simulink model "BaseEngineController_A02"  (Simulink 7.7)
settings: Solver=FixedStepDiscrete  StartTime=0.0  StopTime=10.0  RelTol=1e-3
PreLoadFcn:
  | try, eval([bdroot '_setup']); catch, eval('model_setup', ''); end
InitFcn:
  | try, eval([bdroot '_setup']); catch, eval('model_setup', ''); end
undefined symbols (not defined in the file or in its folder): ECU555, High, motohawk_build_version, motohawk_trunc_name, motohawk_vardec_config_group, motohawk_vardec_path, True
libraries: MotoHawk_lib x1041, AutoDoc_lib x697, motohawk_control_primitives_lib x106, motohawk_engine_lib x18, motohawk_annotations_lib x12, simulink x9, motohawk_control_primitives_motoshare_lib x1, simulink_extras x1
(part 3 of 21)

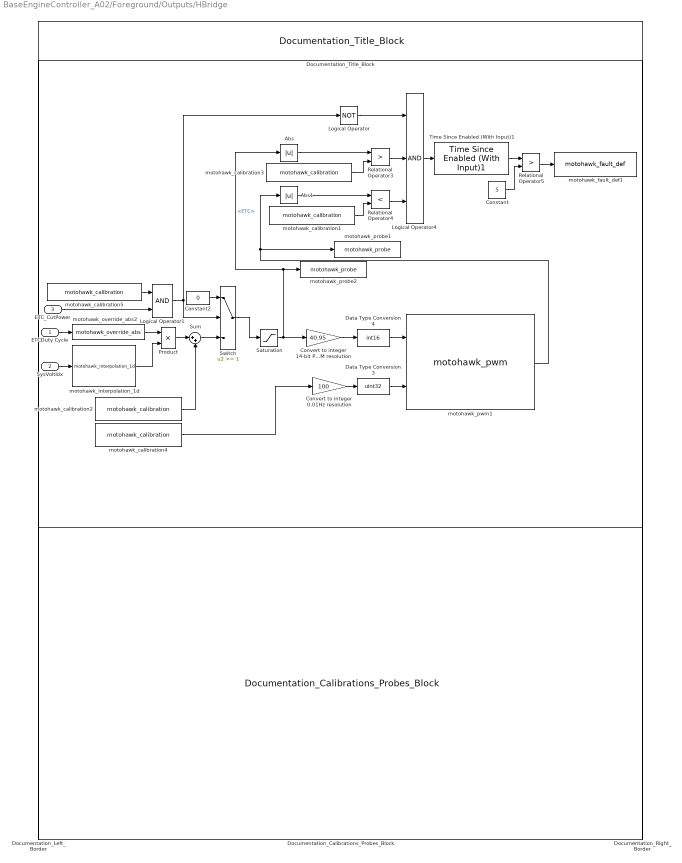
== system BaseEngineController_A02/Foreground/Outputs/HBridge ==
  ETCDuty Cycle : Inport
  SysVoltIdx : Inport  Port=2
  ETC_CutPower : Inport  Port=3
  Abs : Abs
  Abs1 : Abs
  Constant : Constant  Value=5
  Constant2 : Constant  Value=0  SampleTime=-1
  Convert to integer 0.01Hz resolution : Gain  Gain=100  SaturateOnIntegerOverflow=on  OutDataTypeStr=Inherit: Same as input  ParamDataTypeStr=Inherit: Same as input
  Convert to integer 14-bit PWM resolution : Gain  Gain=40.95  SaturateOnIntegerOverflow=on  OutDataTypeStr=Inherit: Same as input  ParamDataTypeStr=Inherit: Same as input
  Data Type Conversion3 : DataTypeConversion  OutDataTypeStr=uint32  SaturateOnIntegerOverflow=on  RndMeth=Zero
  Data Type Conversion4 : DataTypeConversion  OutDataTypeStr=int16  SaturateOnIntegerOverflow=on  RndMeth=Zero
  Documentation_Calibrations_Probes_Block : AutoDoc_lib/Documentation_Calibrations_Probes_Block
  Documentation_Left_Border : AutoDoc_lib/Documentation_Left_Border  layout=Portrait
  Documentation_Right_Border : AutoDoc_lib/Documentation_Right_Border  layout=Portrait
  Documentation_Title_Block : AutoDoc_lib/Documentation_Title_Block
  Logical Operator : Logic  Operator=NOT  AllPortsSameDT=off  OutDataTypeStr=boolean
  Logical Operator1 : Logic  AllPortsSameDT=off  OutDataTypeStr=boolean
  Logical Operator4 : Logic  Inputs=3  AllPortsSameDT=off  OutDataTypeStr=boolean
  Product : Product  SaturateOnIntegerOverflow=on  InputSameDT=on  OutDataTypeStr=Inherit: Same as first input
  Relational Operator3 : RelationalOperator  Operator=>  OutDataTypeStr=boolean
  Relational Operator4 : RelationalOperator  Operator=<  OutDataTypeStr=boolean
  Relational Operator5 : RelationalOperator  Operator=>  OutDataTypeStr=boolean
  Saturation : Saturate  UpperLimit=100  LowerLimit=-100
  Sum : Sum  Inputs=|++  SaturateOnIntegerOverflow=on
  Switch : Switch  Threshold=1
  Time Since Enabled (With Input)1 : motohawk_control_primitives_lib/Time Since Enabled (With Input)1  SystemSampleTime=-1  nam='ETCOpenFaultTimer'  samp_time=-1  init=0  upperlim=16000
  motohawk_calibration1 : MotoHawk_lib/Calibration & Probing Blocks/motohawk_calibration  nam='ETC_I_MIN'  val=5  storage=Calibration  show_more=on  name_source=Use Parameter  data_type=double  read_level=1  write_level=1  ovr_cals=on  view_as=Number  enum_struct={'Disabled', 'Enabled'}  vector_style=Wide Row  use_help_units=on  help="'H-bridge open circuit fault:  ETC current must exceed this when ETC duty cycle is > ETC_OC_MIN, otherwise an open circuit fault may exist.'"  units='mA'  use_header_enum=off  row_header_enum={}  col_header_enum={}  use_min_max=off  min_val=0  max_val=inf  use_precision=off  precision=''  gain=1  offset=0  exp=1  use_mototune_group=on  mototune_group=motohawk_vardec_path(gcb)
  motohawk_calibration2 : MotoHawk_lib/Calibration & Probing Blocks/motohawk_calibration  nam='ETC_Offset'  val=0  storage=Calibration  show_more=on  name_source=Use Parameter  data_type=double  read_level=1  write_level=1  ovr_cals=on  view_as=Number  enum_struct={'Disabled', 'Enabled'}  vector_style=Wide Row  use_help_units=on  help='Offset from engineering units to PWM duty cycle'  units='%'  use_header_enum=off  row_header_enum={}  col_header_enum={}  use_min_max=off  min_val=-inf  max_val=inf  use_precision=off  precision=''  gain=1  offset=0  exp=1  use_mototune_group=on  mototune_group=motohawk_vardec_path(gcb)
  motohawk_calibration3 : MotoHawk_lib/Calibration & Probing Blocks/motohawk_calibration  nam='ETC_OC_MIN'  val=50  storage=Calibration  show_more=on  name_source=Use Parameter  data_type=double  read_level=1  write_level=1  ovr_cals=on  view_as=Number  enum_struct={'Disabled', 'Enabled'}  vector_style=Wide Row  use_help_units=on  help="'H-bridge open circuit fault:  ETC duty cycle must exceed this to enable open circuit fault detection.'"  units='%'  use_header_enum=off  row_header_enum={}  col_header_enum={}  use_min_max=off  min_val=0  max_val=inf  use_precision=off  precision=''  gain=1  offset=0  exp=1  use_mototune_group=on  mototune_group=motohawk_vardec_path(gcb)
  motohawk_calibration4 : MotoHawk_lib/Calibration & Probing Blocks/motohawk_calibration  nam='ETC_Frequency'  val=2500  storage=Calibration  show_more=on  name_source=Use Parameter  data_type=double  read_level=1  write_level=1  ovr_cals=on  view_as=Number  enum_struct={'Disabled', 'Enabled'}  vector_style=Wide Row  use_help_units=on  help='Frequency of PWM output'  units='Hz'  use_header_enum=off  row_header_enum={}  col_header_enum={}  use_min_max=on  min_val=7.63  max_val=inf  use_precision=off  precision=''  gain=1  offset=0  exp=1  use_mototune_group=on  mototune_group=motohawk_vardec_path(gcb)
  motohawk_calibration5 : MotoHawk_lib/Calibration & Probing Blocks/motohawk_calibration  nam='ETC_CutPower_Permitted'  val=1  storage=Calibration  show_more=on  name_source=Use Parameter  data_type=boolean  read_level=1  write_level=1  ovr_cals=on  view_as=Number  enum_struct={'Disabled', 'Enabled'}  vector_style=Wide Row  use_help_units=on  help='Cut power to ETC after ESTOP or long stalled period'  units='flag'  use_header_enum=off  row_header_enum={}  col_header_enum={}  use_min_max=off  min_val=0  max_val=100  use_precision=on  precision='2.3'  gain=1  offset=0  exp=1  use_mototune_group=on  mototune_group=motohawk_vardec_path(gcb)
  motohawk_fault_def1 : MotoHawk_lib/Fault Management Blocks/motohawk_fault_def  nam='ETC_Open_Fault'  mode=Enabled  id=[]  x=25  y=50  input_suspected=on  indeterminate=off  increment_xy=on  downsample_count=1  ovr_cals=on  ovr_actions=on  show_actions=off  action1_name=''  action1_cond=(None)  action2_name=''  action2_cond=(None)  action3_name=''  action3_cond=(None)  action4_name=''  action4_cond=(None)
  motohawk_interpolation_1d : MotoHawk_lib/Lookup Tables/motohawk_interpolation_1d  nam='ETC_VoltageComp'  table_data=ones(1,9)  show_more=on  data_type=Inherit from 'Table Data'  storage=NonAdaptive  read_level=1  write_level=1  ovr_cals=on  use_help=on  help='Gain on ETC duty cycle based on DRVP'  units=''  use_min_max=off  min_val=-inf  max_val=inf  use_precision=off  precision=''  gain=1  offset=0  exp=1  use_links=off  prelookup_block=''  output_name=''  use_mototune_group=on  mototune_group=motohawk_vardec_path(gcb)
  motohawk_override_abs2 : MotoHawk_lib/Calibration & Probing Blocks/motohawk_override_abs  SystemSampleTime=-1  parent_name='ETC'  parent_val=0  show_more=on  name_source=Use Parameter  parent_read_level=1  parent_override_level=1  view_as=Number  enum_struct={'Disabled', 'Enabled'}  use_help_units=on  parent_help='Override result of linear sensor characterization, in engineering units'  parent_units=''  use_min_max=on  parent_min=-inf  parent_max=inf  use_precision=off  parent_precision=''  parent_gain=1  parent_offset=0  parent_exp=1  use_mototune_group=on  parent_group=motohawk_vardec_path(gcb)
  motohawk_probe1 : MotoHawk_lib/Calibration & Probing Blocks/motohawk_probe  nam='ETC_Current'  show_more=on  name_source=Use Parameter  read_level=1  view_as=Number  enum_struct={'Disabled', 'Enabled'}  vector_style=Wide Row  use_help_units=on  help='Current output of ETC duty cycle.'  units='mA'  use_header_enum=off  row_header_enum={}  col_header_enum={}  use_precision=off  precision=''  gain=1  offset=0  exp=1  use_mototune_group=on  mototune_group=motohawk_vardec_path(gcb)
  motohawk_probe2 : MotoHawk_lib/Calibration & Probing Blocks/motohawk_probe  nam='ETC_DutyCycle'  show_more=on  name_source=Use Parameter  read_level=1  view_as=Number  enum_struct={'Disabled', 'Enabled'}  vector_style=Wide Row  use_help_units=on  help='Actual duty cycle of actuator'  units='%'  use_header_enum=off  row_header_enum={}  col_header_enum={}  use_precision=off  precision=''  gain=1  offset=0  exp=1  use_mototune_group=on  mototune_group=motohawk_vardec_path(gcb)
  motohawk_pwm1 : MotoHawk_lib/Analog I//O Blocks/motohawk_pwm  res_name=[]  text_resource=ETC  code_resource=RES_H1  val_resource=1  target=ECU555-80  resource_enum_str=(None)=-1,FUELP=0,ETC=1,HSOL1=2,HSOL2=3,HSOL3=4,HSOL4=5,START=6,OILP=11,FINJ1=12,FINJ2=13,FINJ3=14,FINJ4=15,FINJ5=16,FINJ6=17,AINJ1=18,AINJ2=19,AINJ3=20,AINJ4=21,AINJ5=22,AINJ6=23,TACH=24,EST 1=36,EST 2=37,EST 3=38,EST 4=39,EST 5=40,EST 6=41,EST 7=42,EST 8=43  freq_mode=-1  min_freq=64  use_max_freq=off  max_freq=42949672.96  use_enable=off  use_brake=off  output_current=on  output_fault_status=off  use_child=off  use_vardec=on  nam='ETCPin'  read_level=1  write_level=1  group='System | I/O Pins | Outputs Config'
  wires:
  Abs1/1 -> Relational Operator4/1
  Abs/1 -> Relational Operator3/1
  motohawk_pwm1/1 -> Abs1/1, motohawk_probe1/1
  motohawk_calibration1/1 -> Relational Operator4/2
  motohawk_calibration3/1 -> Relational Operator3/2
  Relational Operator3/1 -> Logical Operator4/2
  Relational Operator4/1 -> Logical Operator4/3
  Logical Operator4/1 -> Time Since Enabled (With Input)1/1
  Time Since Enabled (With Input)1/1 -> Relational Operator5/1
  Constant/1 -> Relational Operator5/2
  Relational Operator5/1 -> motohawk_fault_def1/1
  SysVoltIdx/1 -> motohawk_interpolation_1d/1
  ETCDuty Cycle/1 -> motohawk_override_abs2/1
  motohawk_interpolation_1d/1 -> Product/2
  Saturation/1 -> Abs/1, motohawk_probe2/1, Convert to integer 14-bit PWM resolution/1  "<ETC>"
  motohawk_calibration4/1 -> Convert to integer 0.01Hz resolution/1
  Data Type Conversion3/1 -> motohawk_pwm1/2
  Convert to integer 0.01Hz resolution/1 -> Data Type Conversion3/1
  motohawk_override_abs2/1 -> Product/1
  Product/1 -> Sum/1
  motohawk_calibration2/1 -> Sum/2
  Data Type Conversion4/1 -> motohawk_pwm1/1
  Convert to integer 14-bit PWM resolution/1 -> Data Type Conversion4/1
  Constant2/1 -> Switch/1
  Sum/1 -> Switch/3
  Switch/1 -> Saturation/1
  Logical Operator/1 -> Logical Operator4/1
  Logical Operator1/1 -> Switch/2, Logical Operator/1
  ETC_CutPower/1 -> Logical Operator1/2
  motohawk_calibration5/1 -> Logical Operator1/1

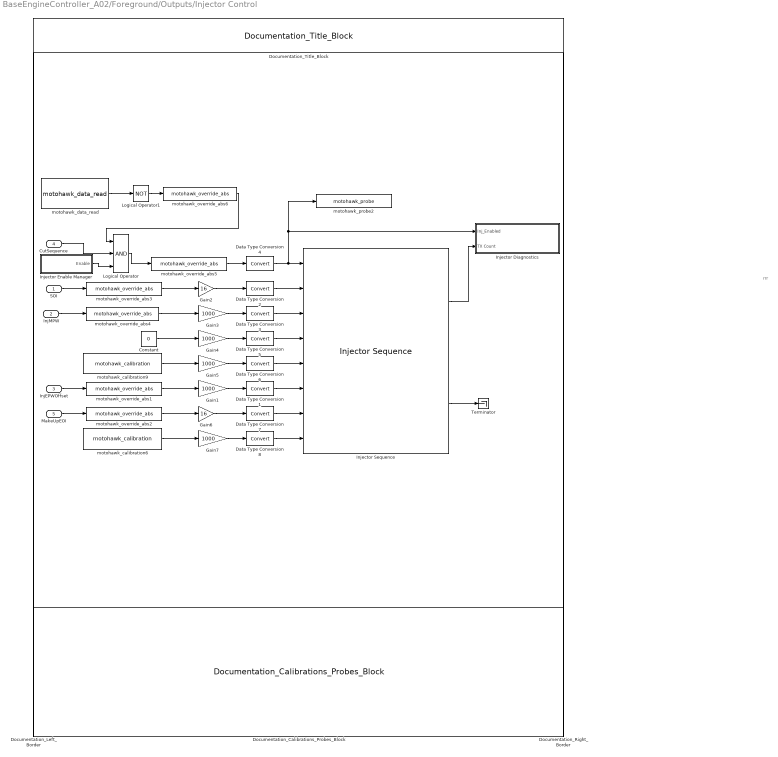
== system BaseEngineController_A02/Foreground/Outputs/Injector Control ==
  note: rrr
  SOI : Inport
  InjMPW : Inport  Port=2
  InjEPWOffset : Inport  Port=3
  CutSequence : Inport  Port=4
  MakeUpEOI : Inport  Port=5
  Constant : Constant  Value=0
  Data Type Conversion{1..8} (8 alike) : DataTypeConversion  SaturateOnIntegerOverflow=on  RndMeth=Zero
  Documentation_Calibrations_Probes_Block : AutoDoc_lib/Documentation_Calibrations_Probes_Block
  Documentation_Left_Border : AutoDoc_lib/Documentation_Left_Border  layout=Portrait
  Documentation_Right_Border : AutoDoc_lib/Documentation_Right_Border  layout=Portrait
  Documentation_Title_Block : AutoDoc_lib/Documentation_Title_Block
  Gain{1,3..5,7} (5 alike) : Gain  Gain=1000  SaturateOnIntegerOverflow=on  OutDataTypeStr=Inherit: Same as input  ParamDataTypeStr=Inherit: Same as input
  Gain2 : Gain  Gain=16  SaturateOnIntegerOverflow=on  OutDataTypeStr=Inherit: Same as input  ParamDataTypeStr=Inherit: Same as input
  Gain6 : Gain  Gain=16  SaturateOnIntegerOverflow=on  OutDataTypeStr=Inherit: Same as input  ParamDataTypeStr=Inherit: Same as input
  Injector Diagnostics : Subsystem  AncestorBlock=MotoHawk_lib/Extra Development Blocks/Injector Diagnostics  mask(Injector Sequence Diagnostics){prefix='Inj', group=motohawk_vardec_path(gcb)}
      mask prompts: group "MotoTune Group"
      mask help:
      | Monitors the output of the Injector Sequence block, and checks for open/short conditions.
      |
      | It outputs vectors for the Open and Short status, appropriate for demuxing and connecting to Fault Definition blocks.
      mask init:
      | lbl = ['Injector\nDiagnostics\n(' prefix ')'];
      mask Display:
      | patch([0 0 1 1], 0.6*[0 0.075 0.075 0], [230/255 0/255 0/255])
      | patch([0 0 1 1], 0.6*[0.025 0.05 0.05 0.025], [150/255 0/255 0/255])
      | patch([0 0 1 1], 0.6*[0 0.025 0.025 0], [0/255 0/255 0/255])
      | 
      | disp(lbl);
  Injector Enable Manager : Subsystem  TreatAsAtomicUnit=on
  Injector Sequence : MotoHawk_lib/Advanced Digital I//O/Injector Sequence  text_resource=FINJ1  code_resource=NONE  val_resource=-1  target=ECU555-80  resource_enum_str=(None)=-1,FINJ1=12,FINJ2=13,FINJ3=14,FINJ4=15,FINJ5=16,FINJ6=17,AINJ1=18,AINJ2=19,AINJ3=20,AINJ4=21,AINJ5=22,AINJ6=23  sequence_type=Wasted (Injector per cylinder & half duration & all cylinders)  behavior=Standard  update_mode=OS  granularity=Fine  use_makeup=off  min_eoi_soi_angle=400  use_diag=on  show_trans_enable=off  min_duration_mode=Static  min_duration=1.5  use_vardec=on  nam='INJ_'  read_level=1  write_level=1  group='System | I/O Pins | Outputs Config'
  Logical Operator : Logic  Inputs=3  AllPortsSameDT=off  OutDataTypeStr=boolean
  Logical Operator1 : Logic  Operator=NOT  AllPortsSameDT=off  OutDataTypeStr=boolean
  Terminator : Terminator
  motohawk_calibration6 : MotoHawk_lib/Calibration & Probing Blocks/motohawk_calibration  nam='MinMakeUpPW'  val=0.1  storage=Calibration  show_more=on  name_source=Use Parameter  data_type=Inherit from 'Default Value'  read_level=1  write_level=1  ovr_cals=on  view_as=Number  enum_struct={'Disabled', 'Enabled'}  vector_style=Wide Row  use_help_units=on  help='Minimum change in injector pulse width that will trigger a make-up pulse.'  units='ms'  use_header_enum=off  row_header_enum={}  col_header_enum={}  use_min_max=off  min_val=-inf  max_val=inf  use_precision=off  precision=''  gain=1  offset=0  exp=1  use_mototune_group=on  mototune_group=motohawk_vardec_path(gcb)
  motohawk_calibration9 : MotoHawk_lib/Calibration & Probing Blocks/motohawk_calibration  nam='InjectorMaxDuration'  val=50  storage=Calibration  show_more=on  name_source=Use Parameter  data_type=Inherit from 'Default Value'  read_level=1  write_level=1  ovr_cals=on  view_as=Number  enum_struct={'Disabled', 'Enabled'}  vector_style=Wide Row  use_help_units=on  help="'Maximum duration for an injector pulse.  Must be a multiple of 5ms'"  units='ms'  use_header_enum=off  row_header_enum={}  col_header_enum={}  use_min_max=off  min_val=-inf  max_val=inf  use_precision=off  precision=''  gain=1  offset=0  exp=1  use_mototune_group=on  mototune_group=motohawk_vardec_path(gcb)
  motohawk_data_read : MotoHawk_lib/Data Storage Blocks/motohawk_data_read  source=Lookup By Name  structure=Scalar  operation=Read scalar from location by index  sz_vec=4  sz_row=2  sz_col=3  typ=boolean  nam='ESTOP'  checkNull=on  struct_inst_name='MyData'  struct_nam='MyStruct'
  motohawk_override_abs1 : MotoHawk_lib/Calibration & Probing Blocks/motohawk_override_abs  SystemSampleTime=-1  parent_name='Elec_PW'  parent_val=0  show_more=on  name_source=Use Parameter  parent_read_level=1  parent_override_level=1  view_as=Number  enum_struct={'Disabled', 'Enabled'}  use_help_units=off  parent_help=''  parent_units=''  use_min_max=off  parent_min=-inf  parent_max=inf  use_precision=off  parent_precision=''  parent_gain=1  parent_offset=0  parent_exp=1  use_mototune_group=on  parent_group=motohawk_vardec_path(gcb)
  motohawk_override_abs2 : MotoHawk_lib/Calibration & Probing Blocks/motohawk_override_abs  SystemSampleTime=-1  parent_name='MakeUp_EOI'  parent_val=0  show_more=on  name_source=Use Parameter  parent_read_level=1  parent_override_level=1  view_as=Number  enum_struct={'Disabled', 'Enabled'}  use_help_units=off  parent_help=''  parent_units=''  use_min_max=off  parent_min=-inf  parent_max=inf  use_precision=off  parent_precision=''  parent_gain=1  parent_offset=0  parent_exp=1  use_mototune_group=on  parent_group=motohawk_vardec_path(gcb)
  motohawk_override_abs3 : MotoHawk_lib/Calibration & Probing Blocks/motohawk_override_abs  SystemSampleTime=-1  parent_name='SOI'  parent_val=0  show_more=on  name_source=Use Parameter  parent_read_level=1  parent_override_level=1  view_as=Number  enum_struct={'Disabled', 'Enabled'}  use_help_units=off  parent_help=''  parent_units=''  use_min_max=off  parent_min=-inf  parent_max=inf  use_precision=off  parent_precision=''  parent_gain=1  parent_offset=0  parent_exp=1  use_mototune_group=on  parent_group=motohawk_vardec_path(gcb)
  motohawk_override_abs4 : MotoHawk_lib/Calibration & Probing Blocks/motohawk_override_abs  SystemSampleTime=-1  parent_name='Mech_PW'  parent_val=0  show_more=on  name_source=Use Parameter  parent_read_level=1  parent_override_level=1  view_as=Number  enum_struct={'Disabled', 'Enabled'}  use_help_units=off  parent_help=''  parent_units=''  use_min_max=off  parent_min=-inf  parent_max=inf  use_precision=off  parent_precision=''  parent_gain=1  parent_offset=0  parent_exp=1  use_mototune_group=on  parent_group=motohawk_vardec_path(gcb)
  motohawk_override_abs5 : MotoHawk_lib/Calibration & Probing Blocks/motohawk_override_abs  SystemSampleTime=-1  parent_name='InjEnable'  parent_val=0  show_more=on  name_source=Use Parameter  parent_read_level=1  parent_override_level=1  view_as=Number  enum_struct={'Disabled', 'Enabled'}  use_help_units=off  parent_help=''  parent_units=''  use_min_max=off  parent_min=-inf  parent_max=inf  use_precision=off  parent_precision=''  parent_gain=1  parent_offset=0  parent_exp=1  use_mototune_group=on  parent_group=motohawk_vardec_path(gcb)
  motohawk_override_abs6 : MotoHawk_lib/Calibration & Probing Blocks/motohawk_override_abs  SystemSampleTime=-1  parent_name='ESTOP_FuelInj_Ovr'  parent_val=0  show_more=on  name_source=Use Parameter  parent_read_level=1  parent_override_level=1  view_as=Number  enum_struct={'Disabled', 'Enabled'}  use_help_units=off  parent_help=''  parent_units=''  use_min_max=off  parent_min=-inf  parent_max=inf  use_precision=off  parent_precision=''  parent_gain=1  parent_offset=0  parent_exp=1  use_mototune_group=on  parent_group=motohawk_vardec_path(gcb)
  motohawk_probe2 : MotoHawk_lib/Calibration & Probing Blocks/motohawk_probe  nam='InjectorsEnabled'  show_more=on  name_source=Use Parameter  read_level=1  view_as=Number  enum_struct={'Disabled', 'Enabled'}  vector_style=Wide Row  use_help_units=on  help='Enable state for injectors.'  units='bool'  use_header_enum=off  row_header_enum={}  col_header_enum={}  use_precision=off  precision=''  gain=1  offset=0  exp=1  use_mototune_group=on  mototune_group=motohawk_vardec_path(gcb)
  wires:
  motohawk_override_abs4/1 -> Gain3/1
  motohawk_override_abs3/1 -> Gain2/1
  motohawk_override_abs2/1 -> Gain6/1
  motohawk_override_abs1/1 -> Gain1/1
  Data Type Conversion8/1 -> Injector Sequence/8
  Gain7/1 -> Data Type Conversion8/1
  Data Type Conversion7/1 -> Injector Sequence/7
  Gain6/1 -> Data Type Conversion7/1
  Data Type Conversion6/1 -> Injector Sequence/5
  Gain5/1 -> Data Type Conversion6/1
  Data Type Conversion5/1 -> Injector Sequence/4
  Gain4/1 -> Data Type Conversion5/1
  Injector Sequence/2 -> Terminator/1
  Injector Sequence/1 -> Injector Diagnostics/2(TX Count)
  Gain1/1 -> Data Type Conversion1/1
  Data Type Conversion1/1 -> Injector Sequence/6
  motohawk_calibration9/1 -> Gain5/1
  Data Type Conversion4/1 -> Injector Diagnostics/1(Inj_Enabled), motohawk_probe2/1, Injector Sequence/1
  motohawk_calibration6/1 -> Gain7/1
  MakeUpEOI/1 -> motohawk_override_abs2/1
  Logical Operator/1 -> motohawk_override_abs5/1
  InjEPWOffset/1 -> motohawk_override_abs1/1
  InjMPW/1 -> motohawk_override_abs4/1
  SOI/1 -> motohawk_override_abs3/1
  Constant/1 -> Gain4/1
  Data Type Conversion3/1 -> Injector Sequence/3
  Data Type Conversion2/1 -> Injector Sequence/2
  Gain2/1 -> Data Type Conversion2/1
  Gain3/1 -> Data Type Conversion3/1
  motohawk_override_abs5/1 -> Data Type Conversion4/1
  Injector Enable Manager/1(Enable) -> Logical Operator/3
  CutSequence/1 -> Logical Operator/2
  motohawk_data_read/1 -> Logical Operator1/1
  Logical Operator1/1 -> motohawk_override_abs6/1
  motohawk_override_abs6/1 -> Logical Operator/1

== system BaseEngineController_A02/Foreground/Outputs/OilPump ==
  State : Inport
  Discrete Actuator - OILP : motohawk_engine_lib/Discrete Actuator Characterization  SystemSampleTime=-1  prefix='OILP'  nam='OILP'  units='bool'  parent_group=motohawk_vardec_path(gcb)  polarity=High-True
  Documentation_Calibrations_Probes_Block : AutoDoc_lib/Documentation_Calibrations_Probes_Block
  Documentation_Left_Border : AutoDoc_lib/Documentation_Left_Border  layout=Portrait
  Documentation_Right_Border : AutoDoc_lib/Documentation_Right_Border  layout=Portrait
  Documentation_Title_Block : AutoDoc_lib/Documentation_Title_Block
  motohawk_dout : MotoHawk_lib/Digital I//O Blocks/motohawk_dout  resource_name=[]  text_resource=OILP  target=ECU555-80  use_diag=off  use_vardec=on  nam='OilPumpPin'  read_level=1  write_level=1  group='System | I/O Pins | Outputs Config'
  wires:
  State/1 -> Discrete Actuator - OILP/1
  Discrete Actuator - OILP/1 -> motohawk_dout/1

== system BaseEngineController_A02/Foreground/Outputs/Oxygen Heater ==
  State : Inport
  Discrete Actuator - Oxygen Heater : motohawk_engine_lib/Discrete Actuator Characterization  SystemSampleTime=-1  prefix='Oxygen_Heater'  nam='O2_Heater'  units='bool'  parent_group=motohawk_vardec_path(gcb)  polarity=High-True
  Documentation_Calibrations_Probes_Block : AutoDoc_lib/Documentation_Calibrations_Probes_Block
  Documentation_Left_Border : AutoDoc_lib/Documentation_Left_Border  layout=Portrait
  Documentation_Right_Border : AutoDoc_lib/Documentation_Right_Border  layout=Portrait
  Documentation_Title_Block : AutoDoc_lib/Documentation_Title_Block
  motohawk_dout : MotoHawk_lib/Digital I//O Blocks/motohawk_dout  resource_name=[]  text_resource=EST 1  target=ECU555-80  use_diag=off  use_vardec=on  nam='O2_Heater'  read_level=1  write_level=1  group='System | I/O Pins | Outputs Config'
  wires:
  State/1 -> Discrete Actuator - Oxygen Heater/1
  Discrete Actuator - Oxygen Heater/1 -> motohawk_dout/1

== system BaseEngineController_A02/Main Power Relay/Main Power Relay/Forced Shutdown Process ==
  ECUP State : Inport
  Documentation_Calibrations_Probes_Block : AutoDoc_lib/Documentation_Calibrations_Probes_Block
  Documentation_Left_Border : AutoDoc_lib/Documentation_Left_Border  layout=Portrait
  Documentation_Right_Border : AutoDoc_lib/Documentation_Right_Border  layout=Portrait
  Documentation_Title_Block : AutoDoc_lib/Documentation_Title_Block
  Logical Operator1 : Logic  Operator=NOT  OutDataTypeStr=boolean
  Logical Operator5 : Logic  Operator=NOT  OutDataTypeStr=boolean
  Post Shutdown two ticks before MPRD off : Subsystem  TreatAsAtomicUnit=on
  Processor Reboot : Subsystem  TreatAsAtomicUnit=on
  Relational Operator1 : RelationalOperator  OutDataTypeStr=boolean  ZeroCross=off  InputSameDT=on
  Save NV Vars one tick before MPRD off : Subsystem  TreatAsAtomicUnit=on
  Shutdown power on ECU565/563 : Subsystem  TreatAsAtomicUnit=on
  Time Since Enabled (With Input)1 : motohawk_control_primitives_lib/Time Since Enabled (With Input)1  SystemSampleTime=-1  nam='ForcedShutdown'  samp_time=-1  init=0  upperlim=16000
  Unit Delay6 : UnitDelay  SampleTime=-1
  Unit Delay7 : UnitDelay  SampleTime=-1
  Unit Delay8 : UnitDelay  SampleTime=-1
  motohawk_calibration1 : MotoHawk_lib/Calibration & Probing Blocks/motohawk_calibration  nam='ForcedShutDownTimerThresh'  val=12  storage=Calibration  show_more=on  name_source=Use Parameter  data_type=Inherit via back propagation  read_level=1  write_level=1  ovr_cals=on  view_as=Number  enum_struct={'Disabled', 'Enabled'}  vector_style=Wide Row  use_help_units=on  help='If ECUP is off for this time and application has not turned off, then force a shutdown routine.'  units='sec'  use_header_enum=off  row_header_enum={}  col_header_enum={}  use_min_max=on  min_val=0  max_val=1023  use_precision=off  precision=''  gain=1  offset=0  exp=1  use_mototune_group=on  mototune_group=motohawk_vardec_config_group(group)
  motohawk_probe1 : MotoHawk_lib/Calibration & Probing Blocks/motohawk_probe  nam='ForcedShutDownTimer'  show_more=on  name_source=Use Parameter  read_level=1  view_as=Number  enum_struct={'Disabled', 'Enabled'}  vector_style=Wide Row  use_help_units=off  help=''  units=''  use_header_enum=off  row_header_enum={}  col_header_enum={}  use_precision=off  precision=''  gain=1  offset=0  exp=1  use_mototune_group=on  mototune_group=group
  wires:
  Logical Operator1/1 -> Time Since Enabled (With Input)1/1
  ECUP State/1 -> Logical Operator1/1
  Unit Delay8/1 -> Processor Reboot/Trigger
  Logical Operator5/1 -> Post Shutdown two ticks before MPRD off/Trigger, Unit Delay6/1
  Unit Delay7/1 -> Shutdown power on ECU565/563/Trigger, Unit Delay8/1
  Unit Delay6/1 -> Unit Delay7/1, Save NV Vars one tick before MPRD off/Trigger
  Relational Operator1/1 -> Logical Operator5/1
  motohawk_calibration1/1 -> Relational Operator1/2
  Time Since Enabled (With Input)1/1 -> Relational Operator1/1, motohawk_probe1/1

== system BaseEngineController_A02/Main Power Relay/Main Power Relay/On Off Delay ==
  note: Delay the rising and falling of a boolean signal
  In : Inport
  Constant1 : Constant  Value=0  OutDataTypeStr=Inherit: Inherit via back propagation
  Constant2 : Constant  Value=on_delay_count  OutDataTypeStr=Inherit: Inherit via back propagation
  Constant3 : Constant  Value=0  OutDataTypeStr=Inherit: Inherit via back propagation
  Constant4 : Constant  Value=off_delay_count  OutDataTypeStr=Inherit: Inherit via back propagation
  Data Type Conversion : DataTypeConversion  SaturateOnIntegerOverflow=on  RndMeth=Zero
  Data Type Conversion1 : DataTypeConversion  SaturateOnIntegerOverflow=on  RndMeth=Zero
  Logical Operator : Logic  Operator=NOT  AllPortsSameDT=off
  Logical Operator1 : Logic  AllPortsSameDT=off
  Logical Operator2 : Logic  AllPortsSameDT=off
  Relational Operator1 : RelationalOperator
  Relational Operator2 : RelationalOperator
  S-R Flip-Flop : simulink_extras/Flip Flops/S-R Flip-Flop  SystemSampleTime=-1  initial_condition=0
  Sum : Sum  Inputs=|++  SaturateOnIntegerOverflow=on
  Sum1 : Sum  Inputs=|++  SaturateOnIntegerOverflow=on
  Switch : Switch  Threshold=1  SaturateOnIntegerOverflow=on
  Switch1 : Switch  Threshold=1  SaturateOnIntegerOverflow=on
  Terminator : Terminator
  Unit Delay : UnitDelay  SampleTime=-1
  Unit Delay1 : UnitDelay  SampleTime=-1
  Out : Outport
  wires:
  Constant2/1 -> Relational Operator1/2
  Switch/1 -> Relational Operator1/1, Unit Delay/1
  Constant1/1 -> Switch/3
  Sum/1 -> Switch/1
  Data Type Conversion/1 -> Sum/1
  Unit Delay/1 -> Sum/2
  In/1 -> Switch/2, Data Type Conversion/1, Logical Operator2/1, Logical Operator/1
  Switch1/1 -> Unit Delay1/1, Relational Operator2/1
  Constant3/1 -> Switch1/3
  Sum1/1 -> Switch1/1
  Data Type Conversion1/1 -> Sum1/1
  Unit Delay1/1 -> Sum1/2
  Logical Operator/1 -> Data Type Conversion1/1, Switch1/2, Logical Operator1/1
  Constant4/1 -> Relational Operator2/2
  S-R Flip-Flop/1 -> Out/1
  S-R Flip-Flop/2 -> Terminator/1
  Relational Operator2/1 -> Logical Operator1/2
  Relational Operator1/1 -> Logical Operator2/2
  Logical Operator2/1 -> S-R Flip-Flop/1
  Logical Operator1/1 -> S-R Flip-Flop/2

== system BaseEngineController_A02/Main Power Relay/Main Power Relay/ShutDown Processes ==
  Manual Reset : Inport
  Input State : Inport  Port=2
  Documentation_Calibrations_Probes_Block : AutoDoc_lib/Documentation_Calibrations_Probes_Block
  Documentation_Left_Border : AutoDoc_lib/Documentation_Left_Border  layout=Portrait
  Documentation_Right_Border : AutoDoc_lib/Documentation_Right_Border  layout=Portrait
  Documentation_Title_Block : AutoDoc_lib/Documentation_Title_Block
  Logical Operator2 : Logic  Operator=OR  AllPortsSameDT=off  OutDataTypeStr=boolean
  Post Shutdown two ticks before MPRD off : Subsystem  TreatAsAtomicUnit=on
  Processor Reboot : Subsystem  TreatAsAtomicUnit=on
  Save NV Vars one tick before MPRD off : Subsystem  TreatAsAtomicUnit=on
  Shutdown power on ECU565/563 : Subsystem  TreatAsAtomicUnit=on
  Unit Delay : UnitDelay  SampleTime=-1
  Unit Delay1 : UnitDelay  SampleTime=-1
  Unit Delay2 : UnitDelay  SampleTime=-1
  motohawk_override_abs : MotoHawk_lib/Calibration & Probing Blocks/motohawk_override_abs  SystemSampleTime=-1  parent_name='MPRD'  parent_val=0  show_more=on  name_source=Use Parameter  parent_read_level=1  parent_override_level=1  view_as=Enumeration  enum_struct={'Off', 'On'}  use_help_units=off  parent_help='Directly Override Main Power Relay'  parent_units='boolean'  use_min_max=off  parent_min=-inf  parent_max=inf  use_precision=off  parent_precision=''  parent_gain=1  parent_offset=0  parent_exp=1  use_mototune_group=on  parent_group=group
  MPRD State : Outport
  wires:
  motohawk_override_abs/1 -> MPRD State/1
  Manual Reset/1 -> Processor Reboot/Enable
  Logical Operator2/1 -> motohawk_override_abs/1
  Unit Delay2/1 -> Logical Operator2/2, Processor Reboot/Trigger
  Input State/1 -> Post Shutdown two ticks before MPRD off/Trigger, Unit Delay/1, Logical Operator2/1
  Unit Delay1/1 -> Unit Delay2/1, Shutdown power on ECU565/563/Trigger
  Unit Delay/1 -> Save NV Vars one tick before MPRD off/Trigger, Unit Delay1/1

== system BaseEngineController_A02/Foreground/Control/ActuatorCharacterization/EGR Characterization ==
  EGR% Request : Inport
  EGR% Pos : Inport  Port=2
  Difference : Sum  Inputs=+-
  EGR Hyst : Subsystem
  Signal Generator : Subsystem
  motohawk_calibration : MotoHawk_lib/Calibration & Probing Blocks/motohawk_calibration  nam='EGR_Hyst'  val=1  storage=Calibration  show_more=off  name_source=Use Parameter  data_type=Inherit from 'Default Value'  read_level=1  write_level=1  ovr_cals=on  view_as=Number  enum_struct={'Disabled', 'Enabled'}  vector_style=Wide Row  use_help_units=off  help=''  units=''  use_header_enum=off  row_header_enum={}  col_header_enum={}  use_min_max=off  min_val=-inf  max_val=inf  use_precision=off  precision=''  gain=1  offset=0  exp=1  use_mototune_group=off  mototune_group=motohawk_vardec_path(gcb)
  motohawk_calibration1 : MotoHawk_lib/Calibration & Probing Blocks/motohawk_calibration  nam='Mode'  val=0  storage=Calibration  show_more=on  name_source=Use Parameter  data_type=uint8  read_level=1  write_level=1  ovr_cals=on  view_as=Enumeration  enum_struct={'PulseWidth', 'DutyCycle', 'Both'}  vector_style=Wide Row  use_help_units=off  help=''  units=''  use_header_enum=off  row_header_enum={}  col_header_enum={}  use_min_max=off  min_val=-inf  max_val=inf  use_precision=off  precision=''  gain=1  offset=0  exp=1  use_mototune_group=off  mototune_group=motohawk_vardec_path(gcb)
  motohawk_interpolation_1d : MotoHawk_lib/Lookup Tables/motohawk_interpolation_1d  nam='EGR_Interpolation_PW'  table_data=[0,0,0,0,0,0,0,0,0,0,0]  show_more=on  data_type=Inherit from 'Table Data'  storage=NonAdaptive  read_level=1  write_level=1  ovr_cals=on  use_help=on  help=''  units='us'  use_min_max=on  min_val=0  max_val=inf  use_precision=off  precision=''  gain=1  offset=0  exp=1  use_links=off  prelookup_block=''  output_name=''  use_mototune_group=off  mototune_group=motohawk_vardec_path(gcb)
  motohawk_interpolation_1d1 : MotoHawk_lib/Lookup Tables/motohawk_interpolation_1d  nam='EGR_Interpolation_DC'  table_data=[0,0,0,0,0,0,0,0,0,0,0]  show_more=on  data_type=Inherit from 'Table Data'  storage=NonAdaptive  read_level=1  write_level=1  ovr_cals=on  use_help=on  help=''  units='%'  use_min_max=on  min_val=0  max_val=100  use_precision=off  precision=''  gain=1  offset=0  exp=1  use_links=off  prelookup_block=''  output_name=''  use_mototune_group=off  mototune_group=motohawk_vardec_path(gcb)
  motohawk_prelookup : MotoHawk_lib/Lookup Tables/motohawk_prelookup  nam='EGR_Prelookup_PW'  breakpoint_data=[-100:20:100]  show_more=on  data_type=Inherit from 'Table Data'  storage=Constant  read_level=1  write_level=1  ovr_cals=on  use_help=on  help=''  units=''  use_min_max=off  min_val=-inf  max_val=inf  use_precision=off  precision=''  gain=1  offset=0  exp=1  use_links=off  input_name=''  use_mototune_group=off  mototune_group=motohawk_vardec_path(gcb)
  motohawk_prelookup1 : MotoHawk_lib/Lookup Tables/motohawk_prelookup  nam='EGR_Prelookup_DC'  breakpoint_data=[-100:20:100]  show_more=off  data_type=Inherit from 'Table Data'  storage=Constant  read_level=1  write_level=1  ovr_cals=on  use_help=off  help=''  units=''  use_min_max=off  min_val=-inf  max_val=inf  use_precision=off  precision=''  gain=1  offset=0  exp=1  use_links=off  input_name=''  use_mototune_group=off  mototune_group=motohawk_vardec_path(gcb)
  EGR : Outport
  wires:
  EGR% Request/1 -> Difference/1
  EGR% Pos/1 -> Difference/2
  Difference/1 -> EGR Hyst/1(Diff)
  motohawk_calibration/1 -> EGR Hyst/2(Hyst)
  EGR Hyst/1(Diff w/Hyst) -> motohawk_prelookup/1, motohawk_prelookup1/1
  motohawk_prelookup/1 -> motohawk_interpolation_1d/1
  motohawk_prelookup1/1 -> motohawk_interpolation_1d1/1
  motohawk_interpolation_1d/1 -> Signal Generator/1(PW)
  motohawk_interpolation_1d1/1 -> Signal Generator/2(DC)
  motohawk_calibration1/1 -> Signal Generator/3(Mode)
  Signal Generator/1(Out) -> EGR/1

== system BaseEngineController_A02/Foreground/Control/ActuatorCharacterization/Electronic Throttle Controller ==
  TPS : Inport
  ETCReq : Inport  Port=2
  Enable : EnablePort
  Constant2 : Constant  Value=0  SampleTime=-1
  Documentation_Calibrations_Probes_Block : AutoDoc_lib/Documentation_Calibrations_Probes_Block
  Documentation_Left_Border : AutoDoc_lib/Documentation_Left_Border  layout=Portrait
  Documentation_Right_Border : AutoDoc_lib/Documentation_Right_Border  layout=Portrait
  Documentation_Title_Block : AutoDoc_lib/Documentation_Title_Block
  ETC Adapt Request : Subsystem
  ETC Sticking Fault : Subsystem
  ETC Test  Manager : Stateflow chart  TreatAsAtomicUnit=on
  ETCStallShutDown : Subsystem
  Logical Operator : Logic  Operator=OR  AllPortsSameDT=off  OutDataTypeStr=boolean
  PID With Damper Terms (Tunable) : Subsystem  AncestorBlock=motohawk_control_primitives_lib_new/PID With Damper Terms (Tunable)  mask(PID with Damper Terms (Tunable)){nam='Electronic Throttle Control', prefix='ETC', samp_time=-1, units='%', grp=motohawk_vardec_path(gcb)}
      mask prompts: nam "Name"; samp_time "Sample Time"; grp "MotoTune Group String"
      mask help:
      | This block wraps a 'PID With Damper Terms' block to provide calibrations and probes for most parameters.
      |
      | If 'Sample Time' is -1, then extra code and RAM will be generated to dynamically calculate dt.
      mask Display:
      | patch([0 0 1 1], [0 0.075 0.075 0], [230/255 0/255 0/255])
      | patch([0 0 1 1], [0.025 0.05 0.05 0.025], [150/255 0/255 0/255])
      | patch([0 0 1 1], [0 0.025 0.025 0], [0/255 0/255 0/255])
      | 
      | disp([nam '\n(PIDD)']);
  Relational Operator1 : RelationalOperator  Operator=<  ZeroCross=off  InputSameDT=on
  Relational Operator2 : RelationalOperator  Operator=>  ZeroCross=off  InputSameDT=on
  Relational Operator3 : RelationalOperator  Operator=<  ZeroCross=off  InputSameDT=on
  Relational Operator5 : RelationalOperator  Operator=<  ZeroCross=off  InputSameDT=on
  Reset TasksComplete To True : Subsystem  TreatAsAtomicUnit=on
  Setpoint Manager : Subsystem
  State  Manager : Subsystem
  Switch : Switch  Threshold=1
  Unit Delay : UnitDelay  SampleTime=-1
  motohawk_calibration3 : MotoHawk_lib/Calibration & Probing Blocks/motohawk_calibration  nam='ETC_SpringTestTimerThresh'  val=2  storage=Calibration  show_more=on  name_source=Use Parameter  data_type=double  read_level=1  write_level=1  ovr_cals=on  view_as=Number  enum_struct={'Disabled', 'Enabled'}  vector_style=Wide Row  use_help_units=on  help='During spring test at shutdown, TPS must fall to 0% within this time to prevent fault.'  units='s'  use_header_enum=off  row_header_enum={}  col_header_enum={}  use_min_max=on  min_val=-inf  max_val=inf  use_precision=off  precision=''  gain=1  offset=0  exp=1  use_mototune_group=on  mototune_group=motohawk_vardec_path(gcb)
  motohawk_calibration4 : MotoHawk_lib/Calibration & Probing Blocks/motohawk_calibration  nam='ETC_ShutdownLoAdaptError'  val=-10  storage=Calibration  show_more=on  name_source=Use Parameter  data_type=double  read_level=1  write_level=1  ovr_cals=on  view_as=Number  enum_struct={'Disabled', 'Enabled'}  vector_style=Wide Row  use_help_units=on  help='Drive ETC closed until this error has been achieved in order to catch lo side adapt point.'  units='%'  use_header_enum=off  row_header_enum={}  col_header_enum={}  use_min_max=on  min_val=-inf  max_val=inf  use_precision=off  precision=''  gain=1  offset=0  exp=1  use_mototune_group=on  mototune_group=motohawk_vardec_path(gcb)
  motohawk_calibration5 : MotoHawk_lib/Calibration & Probing Blocks/motohawk_calibration  nam='ETC_SpringTestHiThresh'  val=75  storage=Calibration  show_more=on  name_source=Use Parameter  data_type=double  read_level=1  write_level=1  ovr_cals=on  view_as=Number  enum_struct={'Disabled', 'Enabled'}  vector_style=Wide Row  use_help_units=on  help='Before initiating spring test during shutdown, open the ETC to this setpoint. When achieved, the ETC controller will release the ETC to have the spring close the valve.'  units='%'  use_header_enum=off  row_header_enum={}  col_header_enum={}  use_min_max=on  min_val=-inf  max_val=inf  use_precision=off  precision=''  gain=1  offset=0  exp=1  use_mototune_group=on  mototune_group=motohawk_vardec_path(gcb)
  motohawk_calibration6 : MotoHawk_lib/Calibration & Probing Blocks/motohawk_calibration  nam='ETC_SpringTestLoThresh'  val=40  storage=Calibration  show_more=on  name_source=Use Parameter  data_type=double  read_level=1  write_level=1  ovr_cals=on  view_as=Number  enum_struct={'Disabled', 'Enabled'}  vector_style=Wide Row  use_help_units=on  help='Spring test timer will measure time of travel between ETC_SpringTestHiThresh and ETC_SpringTestLoThresh.'  units='%'  use_header_enum=off  row_header_enum={}  col_header_enum={}  use_min_max=on  min_val=-inf  max_val=inf  use_precision=off  precision=''  gain=1  offset=0  exp=1  use_mototune_group=on  mototune_group=motohawk_vardec_path(gcb)
  motohawk_data_def : MotoHawk_lib/Data Storage Blocks/motohawk_data_def  nam='SpringTestTime'  container_nam=''  data=0  typ=Inherit from 'Initial Value'  struct_nam='MyStruct'  storage=NonVolatile  outputRef=off  use_vardec=on  window=Display  read_level=1  write_level=1  ovr_cals=on  view_as=Number  enum_struct={'Disabled', 'Enabled'}  vector_style=Wide Row  use_help_units=on  help='Time for ETC to close from 80% to 40% at 0% duty cycle.'  units='s'  use_header_enum=off  row_header_enum={}  col_header_enum={}  use_min_max=off  min_val=-inf  max_val=inf  use_precision=off  precision=''  gain=1  offset=0  exp=1  use_mototune_group=on  mototune_group=motohawk_vardec_path(gcb)  eval_name=SpringTestTime  data_stores=motohawk_sfun_data_def_data_stores  struct_instances=motohawk_sfun_data_def_struct_instances  vardecs=motohawk_sfun_data_def_vardecs
  motohawk_data_read : MotoHawk_lib/Data Storage Blocks/motohawk_data_read  source=Lookup By Name  structure=Scalar  operation=Read scalar from location by index  sz_vec=4  sz_row=2  sz_col=3  typ=boolean  nam='ETCStallStateShutdown'  checkNull=on  struct_inst_name='Struct1'  struct_nam='MyStruct'
  motohawk_data_write : MotoHawk_lib/Data Storage Blocks/motohawk_data_write  source=Lookup By Name  structure=Scalar  operation=Write entire data structure at once  sz_vec=-1  sz_row=3  sz_col=2  typ=double  nam='SpringTestTime'  checkNull=off  write_read_only=off  struct_inst_name='Struct1'  struct_name='MyStruct'
  motohawk_fault_def : MotoHawk_lib/Fault Management Blocks/motohawk_fault_def  nam='ETCSpringTest'  mode=Sticky  id=[]  x=1  y=2  input_suspected=on  indeterminate=off  increment_xy=on  downsample_count=1  ovr_cals=on  ovr_actions=on  show_actions=off  action1_name=''  action1_cond=(None)  action2_name=''  action2_cond=(None)  action3_name=''  action3_cond=(None)  action4_name=''  action4_cond=(None)
  ETC : Outport
  ETC_TestActive : Outport  Port=2
  wires:
  motohawk_data_read/1 -> PID With Damper Terms (Tunable)/3(ResetITerm)
  TPS/1 -> Relational Operator2/1, Relational Operator3/1, PID With Damper Terms (Tunable)/1(Actual), Setpoint Manager/1(TPS Position)
  ETCReq/1 -> Setpoint Manager/2(Controller Setpoint)
  ETC Test  Manager/2(DC_Override) -> Logical Operator/1
  Switch/1 -> ETC/1
  State  Manager/2(ECUP Timer) -> ETC Test  Manager/2(Timer)
  ETC Test  Manager/3(TestComplete) -> Reset TasksComplete To True/Trigger
  Relational Operator1/1 -> motohawk_fault_def/1
  Constant2/1 -> Switch/1
  Logical Operator/1 -> ETC_TestActive/1, Switch/2
  PID With Damper Terms (Tunable)/1(Out) -> Switch/3
  Relational Operator2/1 -> ETC Test  Manager/4(TPSHiThresh)
  Relational Operator3/1 -> ETC Test  Manager/5(TPSLoThresh)
  ETC Test  Manager/1(TestTime) -> Relational Operator1/2, motohawk_data_write/1
  motohawk_calibration3/1 -> Relational Operator1/1
  Setpoint Manager/1(ETC_Setpoint) -> PID With Damper Terms (Tunable)/2(SetPoint)
  ETC Test  Manager/4(SetpointMode) -> ETC Adapt Request/1(ETC Setpoint Mode), Setpoint Manager/3(Setpoint Mode), ETC Sticking Fault/2(ETC Setpoint Mode)
  Relational Operator5/1 -> ETC Test  Manager/3(LowAdaptError)
  Unit Delay/1 -> Relational Operator5/1
  State  Manager/1(ECUP State) -> ETC Test  Manager/1(ECUP_Off)
  motohawk_calibration4/1 -> Relational Operator5/2
  motohawk_calibration5/1 -> Relational Operator2/2
  motohawk_calibration6/1 -> Relational Operator3/2
  Setpoint Manager/2(ETC_Error) -> ETC Sticking Fault/1(ETC Error), Unit Delay/1, ETC Adapt Request/2(ETC Error)
  ETCStallShutDown/1(State) -> Logical Operator/2

== system BaseEngineController_A02/Foreground/Control/ActuatorCharacterization/Injector Characterization ==
  FPC [mg] : Inport
  FuelMult : Inport  Port=2
  BaroPress [kPa] : Inport  Port=3
  MAP [kPa] : Inport  Port=4
  SysVolt09Idx : Inport  Port=5
  RPM : Inport  Port=6
  Constant : Constant  Value=60*1000*2
  DeltaPressureIndex : Subsystem
  Divide : Product  Inputs=*/
  Documentation_Calibrations_Probes_Block : AutoDoc_lib/Documentation_Calibrations_Probes_Block
  Documentation_Left_Border : AutoDoc_lib/Documentation_Left_Border  layout=Portrait
  Documentation_Right_Border : AutoDoc_lib/Documentation_Right_Border  layout=Portrait
  Documentation_Title_Block : AutoDoc_lib/Documentation_Title_Block
  Fuel Inj Offsets : Subsystem
  Injector Gain [ms/mg] : MotoHawk_lib/Calibration & Probing Blocks/motohawk_calibration  nam='InjGain'  val=0.351  storage=Calibration  show_more=on  name_source=Use Parameter  data_type=Inherit from 'Default Value'  read_level=1  write_level=1  ovr_cals=on  view_as=Number  enum_struct={'Disabled', 'Enabled'}  vector_style=Wide Row  use_help_units=on  help='Gain value used in the linear transformation of FPC to mechanical pulsewidth.'  units='ms/mg'  use_header_enum=off  row_header_enum={}  col_header_enum={}  use_min_max=off  min_val=0  max_val=inf  use_precision=off  precision='2.4'  gain=1  offset=0  exp=1  use_mototune_group=on  mototune_group=motohawk_vardec_path(gcb)
  Injector Offset [ms] : MotoHawk_lib/Calibration & Probing Blocks/motohawk_calibration  nam='InjOffset'  val=-0.05  storage=Calibration  show_more=on  name_source=Use Parameter  data_type=Inherit from 'Default Value'  read_level=1  write_level=1  ovr_cals=on  view_as=Number  enum_struct={'Disabled', 'Enabled'}  vector_style=Wide Row  use_help_units=on  help='Offset value used in the linear transformation of FPC to mechanical pulsewidth.'  units='ms'  use_header_enum=off  row_header_enum={}  col_header_enum={}  use_min_max=off  min_val=-inf  max_val=inf  use_precision=off  precision='3.4'  gain=1  offset=0  exp=1  use_mototune_group=on  mototune_group=motohawk_vardec_path(gcb)
  MinMax : MinMax  Inputs=2
  Mux1 : Mux  Inputs=3
  Product2 : Product  SaturateOnIntegerOverflow=on  InputSameDT=on  OutDataTypeStr=Inherit: Same as first input
  Product3 : Product  Inputs=3  SaturateOnIntegerOverflow=on  InputSameDT=on  OutDataTypeStr=Inherit: Same as first input
  Regulated Gauge Fuel Pressure [kPa] : MotoHawk_lib/Calibration & Probing Blocks/motohawk_calibration  nam='GaugeFuelPress'  val=343  storage=Calibration  show_more=on  name_source=Use Parameter  data_type=Inherit from 'Default Value'  read_level=1  write_level=1  ovr_cals=on  view_as=Number  enum_struct={'Disabled', 'Enabled'}  vector_style=Wide Row  use_help_units=on  help='Fuel pressure of fuel rail.'  units='kPa'  use_header_enum=off  row_header_enum={}  col_header_enum={}  use_min_max=on  min_val=0  max_val=inf  use_precision=off  precision=''  gain=1  offset=0  exp=1  use_mototune_group=on  mototune_group=motohawk_vardec_path(gcb)
  Saturation : Saturate  UpperLimit=inf  LowerLimit=.01
  Sum : Sum  Inputs=|++  SaturateOnIntegerOverflow=on  InputSameDT=on
  Sum1 : Sum  Inputs=|+-  SaturateOnIntegerOverflow=on
  Sum2 : Sum  Inputs=|-+  SaturateOnIntegerOverflow=on
  motohawk_interpolation_1d1 : MotoHawk_lib/Lookup Tables/motohawk_interpolation_1d  nam='InjTurnOn'  table_data=[1.495 1.293 1.155 1.05 0.968 0.898 0.865 0.823 0.743]  show_more=on  data_type=Inherit from 'Table Data'  storage=NonAdaptive  read_level=1  write_level=1  ovr_cals=on  use_help=on  help='Injector turn on time.'  units='ms'  use_min_max=off  min_val=-inf  max_val=inf  use_precision=off  precision='2.3'  gain=1  offset=0  exp=1  use_links=off  prelookup_block=''  output_name=''  use_mototune_group=on  mototune_group=motohawk_vardec_path(gcb)
  motohawk_interpolation_1d3 : MotoHawk_lib/Lookup Tables/motohawk_interpolation_1d  nam='InjdPComp'  table_data=[1 1 1 1 1 1 1 1 1]  show_more=on  data_type=Inherit from 'Table Data'  storage=NonAdaptive  read_level=1  write_level=1  ovr_cals=on  use_help=on  help='Injector characterization compenastion based on dP across injector.'  units=''  use_min_max=on  min_val=-inf  max_val=inf  use_precision=on  precision='2.3'  gain=1  offset=0  exp=1  use_links=off  prelookup_block=''  output_name=''  use_mototune_group=on  mototune_group=motohawk_vardec_path(gcb)
  motohawk_interpolation_1d4 : MotoHawk_lib/Lookup Tables/motohawk_interpolation_1d  nam='InjTurnOff'  table_data=[0.585 0.583333 0.581667 0.58 0.579167 0.578333 0.576667 0.575833 0.575]  show_more=on  data_type=Inherit from 'Table Data'  storage=NonAdaptive  read_level=1  write_level=1  ovr_cals=on  use_help=on  help='Injector turn off time'  units='ms'  use_min_max=on  min_val=-inf  max_val=inf  use_precision=on  precision='2.3'  gain=1  offset=0  exp=1  use_links=off  prelookup_block=''  output_name=''  use_mototune_group=on  mototune_group=motohawk_vardec_path(gcb)
  motohawk_probe1 : MotoHawk_lib/Calibration & Probing Blocks/motohawk_probe  nam='InjEPW'  show_more=on  name_source=Use Parameter  read_level=1  view_as=Number  enum_struct={'Disabled', 'Enabled'}  vector_style=Wide Row  use_help_units=on  help=''  units=''  use_header_enum=off  row_header_enum={}  col_header_enum={}  use_precision=off  precision=''  gain=1  offset=0  exp=1  use_mototune_group=on  mototune_group=motohawk_vardec_path(gcb)
  motohawk_probe3 : MotoHawk_lib/Calibration & Probing Blocks/motohawk_probe  nam='InjMPW'  show_more=on  name_source=Use Parameter  read_level=1  view_as=Number  enum_struct={'Disabled', 'Enabled'}  vector_style=Wide Row  use_help_units=on  help=''  units=''  use_header_enum=off  row_header_enum={}  col_header_enum={}  use_precision=off  precision=''  gain=1  offset=0  exp=1  use_mototune_group=on  mototune_group=motohawk_vardec_path(gcb)
  motohawk_probe4 : MotoHawk_lib/Calibration & Probing Blocks/motohawk_probe  nam='MaxAllowableMPW'  show_more=on  name_source=Use Parameter  read_level=1  view_as=Number  enum_struct={'Disabled', 'Enabled'}  vector_style=Wide Row  use_help_units=on  help=''  units=''  use_header_enum=off  row_header_enum={}  col_header_enum={}  use_precision=off  precision=''  gain=1  offset=0  exp=1  use_mototune_group=on  mototune_group=motohawk_vardec_path(gcb)
  MPW [ms] : Outport
  EPWOffset [ms] : Outport  Port=2
  wires:
  DeltaPressureIndex/1(Index) -> motohawk_interpolation_1d3/1, motohawk_interpolation_1d4/1
  FPC [mg]/1 -> Fuel Inj Offsets/1(Fuel In)
  Injector Gain [ms/mg]/1 -> Product2/2
  Injector Offset [ms]/1 -> Sum/2
  SysVolt09Idx/1 -> motohawk_interpolation_1d1/1
  Sum/1 -> Product3/2
  motohawk_interpolation_1d1/1 -> Sum2/2
  motohawk_interpolation_1d4/1 -> Sum2/1
  Mux1/1 -> DeltaPressureIndex/1(Inputs)
  Regulated Gauge Fuel Pressure [kPa]/1 -> Mux1/1
  BaroPress [kPa]/1 -> Mux1/2
  MAP [kPa]/1 -> Mux1/3
  Sum2/1 -> EPWOffset [ms]/1, Sum1/2, motohawk_probe1/1
  Product2/1 -> Sum/1
  Fuel Inj Offsets/1(Fuel Out) -> Product2/1
  Constant/1 -> Divide/1
  Product3/1 -> MinMax/1
  MinMax/1 -> motohawk_probe3/1, MPW [ms]/1
  Sum1/1 -> MinMax/2, motohawk_probe4/1
  Divide/1 -> Sum1/1
  RPM/1 -> Saturation/1
  Saturation/1 -> Divide/2
  motohawk_interpolation_1d3/1 -> Product3/3
  FuelMult/1 -> Product3/1
